simulink library "Renault_Library"  (Simulink 9.2)
settings: Solver=ode3  StartTime=0.0  StopTime=10.0  FixedStep=0.020  RelTol=1e-3  EnableMultiTasking=on
undefined symbols (not defined in the file or in its folder): ABC, AssystemProposal, b, chkval, CoeffA_C, CoeffB_C, CoeffMean_C, ConfTime_C, DiagDelay_C, DividePercent, FailureConfActive_C, FallingEdgeDuration_C, HighLevelThresholdVoltage_C, HighLevelVoltage_C, HighLevelVoltageTunable_C, InitValue_C, InputVoltRangeMax_C, InputVoltRangeMin_C, LowActiveContact_C, LowLevelThresholdVoltage_C, LowLevelVoltage_C, MaxOperatingVoltage_C, MinOperatingVoltage_C, mV2V_conversion, OUT1, OutOfRangeHigh_C, OutOfRangeLow_C, PinSupplySaturationMax, PinSupplySaturationMin, Res1Value_C, Res2Value_C, Res3Value_C, RisingEdgeDuration_C, RSA_CANSignalFailMonitoring, SampleTime_C, service14Table, service19Table, service22Table, service24Table, service28Table, service2ETable, service2FTable, service31Table, service87Table, SizeXStateDID2FTable, SizeXTable14, SizeXTable19, SizeXTable22, SizeXTable24, SizeXTable28, SizeXTable2E, SizeXTable2F, SizeXTable31, SizeXTable87, SizeYStateDID2FTable, SizeYTable14, SizeYTable19, SizeYTable22, SizeYTable24, SizeYTable28 ... (+22)
undefined symbols (not defined in the file): BatteryVoltage_ActivationThreshold_C, FrameStatus_AbsentValue_C, FrameStatus_ConfAbsentValue_C, MemThreshold_C, NAMEOFTHEMODEL_SAMPLE_TIME_CST, NbAbs1_C, NbConfAbs1_C, NbConfPres1_C, NbPres1_C, NOMDUSIGNAL_FaultConfTime_C, NOMDUSIGNAL_FaultRehabTime_C, NOMDUSIGNAL_switchvalue_C, NOMDUSIGNAL_unavailablevalue_C, PeriodFrame1_C, ResetTime_C, WakeUpTime_C
libraries: $bdroot x112, simulink x75
(part 25 of 32)

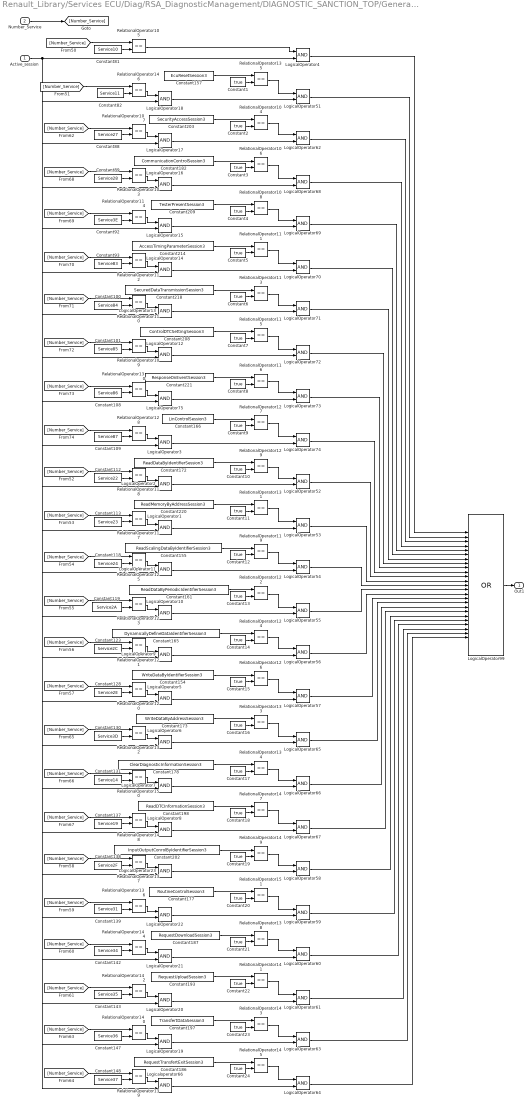
== system Renault_Library/Services ECU/Diag/RSA_DiagnosticManagement/DIAGNOSTIC_SANCTION_TOP/GeneralService/Service_Supported_In_Active_Session/SubsystemExtendedSession ==
  Active_session : Inport
  Number_Service : Inport  Port=2
  Constant{1..24} (24 alike) : Constant  Value=true  OutDataTypeStr=boolean  SampleTime=-1
  Constant100 : Constant  Value=Service84  OutDataTypeStr=Inherit: Inherit via back propagation  SampleTime=-1
  Constant101 : Constant  Value=Service85  OutDataTypeStr=Inherit: Inherit via back propagation  SampleTime=-1
  Constant108 : Constant  Value=Service86  OutDataTypeStr=Inherit: Inherit via back propagation  SampleTime=-1
  Constant109 : Constant  Value=Service87  OutDataTypeStr=Inherit: Inherit via back propagation  SampleTime=-1
  Constant112 : Constant  Value=Service22  OutDataTypeStr=Inherit: Inherit via back propagation  SampleTime=-1
  Constant113 : Constant  Value=Service23  OutDataTypeStr=Inherit: Inherit via back propagation  SampleTime=-1
  Constant118 : Constant  Value=Service24  OutDataTypeStr=Inherit: Inherit via back propagation  SampleTime=-1
  Constant119 : Constant  Value=Service2A  OutDataTypeStr=Inherit: Inherit via back propagation  SampleTime=-1
  Constant123 : Constant  Value=Service2C  OutDataTypeStr=Inherit: Inherit via back propagation  SampleTime=-1
  Constant128 : Constant  Value=Service2E  OutDataTypeStr=Inherit: Inherit via back propagation  SampleTime=-1
  Constant130 : Constant  Value=Service3D  OutDataTypeStr=Inherit: Inherit via back propagation  SampleTime=-1
  Constant131 : Constant  Value=Service14  OutDataTypeStr=Inherit: Inherit via back propagation  SampleTime=-1
  Constant137 : Constant  Value=Service19  OutDataTypeStr=Inherit: Inherit via back propagation  SampleTime=-1
  Constant138 : Constant  Value=Service2F  OutDataTypeStr=Inherit: Inherit via back propagation  SampleTime=-1
  Constant139 : Constant  Value=Service31  OutDataTypeStr=Inherit: Inherit via back propagation  SampleTime=-1
  Constant142 : Constant  Value=Service34  OutDataTypeStr=Inherit: Inherit via back propagation  SampleTime=-1
  Constant143 : Constant  Value=Service35  OutDataTypeStr=Inherit: Inherit via back propagation  SampleTime=-1
  Constant147 : Constant  Value=Service36  OutDataTypeStr=Inherit: Inherit via back propagation  SampleTime=-1
  Constant148 : Constant  Value=Service37  OutDataTypeStr=Inherit: Inherit via back propagation  SampleTime=-1
  Constant154 : Constant  Value=WriteDataByIdentifierSession3  OutDataTypeStr=Inherit: Inherit via back propagation  SampleTime=-1
  Constant155 : Constant  Value=ReadScalingDataByIdentifierSession3  OutDataTypeStr=Inherit: Inherit via back propagation  SampleTime=-1
  Constant157 : Constant  Value=EcuResetSession3  OutDataTypeStr=Inherit: Inherit via back propagation  SampleTime=-1
  Constant161 : Constant  Value=ReadDataByPeriodicIdentifierSession3  OutDataTypeStr=Inherit: Inherit via back propagation  SampleTime=-1
  Constant165 : Constant  Value=DynamicallyDefineDataIdentifierSession3  OutDataTypeStr=Inherit: Inherit via back propagation  SampleTime=-1
  Constant166 : Constant  Value=LinControlSession3  OutDataTypeStr=Inherit: Inherit via back propagation  SampleTime=-1
  Constant172 : Constant  Value=ReadDataByIdentifierSession3  OutDataTypeStr=Inherit: Inherit via back propagation  SampleTime=-1
  Constant173 : Constant  Value=WriteDataByAddressSession3  OutDataTypeStr=Inherit: Inherit via back propagation  SampleTime=-1
  Constant177 : Constant  Value=RoutineControlSession3  OutDataTypeStr=Inherit: Inherit via back propagation  SampleTime=-1
  Constant178 : Constant  Value=ClearDiagnosticInformationSession3  OutDataTypeStr=Inherit: Inherit via back propagation  SampleTime=-1
  Constant182 : Constant  Value=CommunicationControlSession3  OutDataTypeStr=Inherit: Inherit via back propagation  SampleTime=-1
  Constant186 : Constant  Value=RequestTransfertExitSession3  OutDataTypeStr=Inherit: Inherit via back propagation  SampleTime=-1
  Constant187 : Constant  Value=RequestDownloadSession3  OutDataTypeStr=Inherit: Inherit via back propagation  SampleTime=-1
  Constant193 : Constant  Value=RequestUploadSession3  OutDataTypeStr=Inherit: Inherit via back propagation  SampleTime=-1
  Constant197 : Constant  Value=TransfertDataSession3  OutDataTypeStr=Inherit: Inherit via back propagation  SampleTime=-1
  Constant198 : Constant  Value=ReadDTCInformationSession3  OutDataTypeStr=Inherit: Inherit via back propagation  SampleTime=-1
  Constant202 : Constant  Value=InputOutputConrolByIdentifierSession3  OutDataTypeStr=Inherit: Inherit via back propagation  SampleTime=-1
  Constant203 : Constant  Value=SecurityAccessSession3  OutDataTypeStr=Inherit: Inherit via back propagation  SampleTime=-1
  Constant208 : Constant  Value=ControlDTCSettingSession3  OutDataTypeStr=Inherit: Inherit via back propagation  SampleTime=-1
  Constant209 : Constant  Value=TesterPresentSession3  OutDataTypeStr=Inherit: Inherit via back propagation  SampleTime=-1
  Constant214 : Constant  Value=AccessTimingParameterSession3  OutDataTypeStr=Inherit: Inherit via back propagation  SampleTime=-1
  Constant218 : Constant  Value=SecuredDataTransmissionSession3  OutDataTypeStr=Inherit: Inherit via back propagation  SampleTime=-1
  Constant220 : Constant  Value=ReadMemoryByAddressSession3  OutDataTypeStr=Inherit: Inherit via back propagation  SampleTime=-1
  Constant221 : Constant  Value=ResponseOnEventSession3  OutDataTypeStr=Inherit: Inherit via back propagation  SampleTime=-1
  Constant81 : Constant  Value=Service10  OutDataTypeStr=Inherit: Inherit via back propagation  SampleTime=-1
  Constant82 : Constant  Value=Service11  OutDataTypeStr=Inherit: Inherit via back propagation  SampleTime=-1
  Constant88 : Constant  Value=Service27  OutDataTypeStr=Inherit: Inherit via back propagation  SampleTime=-1
  Constant89 : Constant  Value=Service28  OutDataTypeStr=Inherit: Inherit via back propagation  SampleTime=-1
  Constant92 : Constant  Value=Service3E  OutDataTypeStr=Inherit: Inherit via back propagation  SampleTime=-1
  Constant93 : Constant  Value=Service83  OutDataTypeStr=Inherit: Inherit via back propagation  SampleTime=-1
  LogicalOperator{1..23,51..75} (48 alike) : Logic  OutDataTypeStr=boolean
  LogicalOperator99 : Logic  Operator=OR  Inputs=25  OutDataTypeStr=boolean
  Logicaloperator66 : Logic  OutDataTypeStr=boolean
  RelationalOperator{103..151} (49 alike) : RelationalOperator  Operator===  OutDataTypeStr=boolean  RndMeth=Nearest  InputSameDT=on
  Out1 : Outport
  wires:
  Constant81/1 -> RelationalOperator105/2
  Active_session/1 -> LogicalOperator19/2, LogicalOperator20/2, LogicalOperator21/2, LogicalOperator22/2, LogicalOperator23/2, LogicalOperator8/2, LogicalOperator7/2, Logicaloperator66/2, LogicalOperator6/2, LogicalOperator5/2, LogicalOperator9/2, LogicalOperator10/2, LogicalOperator11/2, LogicalOperator1/2, LogicalOperator2/2, LogicalOperator3/2, LogicalOperator75/2, LogicalOperator12/2, LogicalOperator13/2, LogicalOperator14/2, LogicalOperator15/2, LogicalOperator16/2, LogicalOperator17/2, LogicalOperator18/2, LogicalOperator4/2
  RelationalOperator105/1 -> LogicalOperator4/1
  [Number_Service] -> RelationalOperator105/1
  Constant82/1 -> RelationalOperator146/2
  Constant157/1 -> RelationalOperator135/1
  Constant1/1 -> RelationalOperator135/2
  RelationalOperator135/1 -> LogicalOperator51/1
  LogicalOperator18/1 -> LogicalOperator51/2
  RelationalOperator146/1 -> LogicalOperator18/1
  [Number_Service] -> RelationalOperator146/1
  Constant88/1 -> RelationalOperator107/2
  Constant203/1 -> RelationalOperator104/1
  Constant2/1 -> RelationalOperator104/2
  RelationalOperator104/1 -> LogicalOperator62/1
  LogicalOperator17/1 -> LogicalOperator62/2
  RelationalOperator107/1 -> LogicalOperator17/1
  [Number_Service] -> RelationalOperator107/1
  Constant89/1 -> RelationalOperator103/2
  Constant182/1 -> RelationalOperator106/1
  Constant3/1 -> RelationalOperator106/2
  RelationalOperator106/1 -> LogicalOperator68/1
  LogicalOperator16/1 -> LogicalOperator68/2
  RelationalOperator103/1 -> LogicalOperator16/1
  [Number_Service] -> RelationalOperator103/1
  Constant92/1 -> RelationalOperator114/2
  Constant209/1 -> RelationalOperator108/1
  Constant4/1 -> RelationalOperator108/2
  RelationalOperator108/1 -> LogicalOperator69/1
  LogicalOperator15/1 -> LogicalOperator69/2
  RelationalOperator114/1 -> LogicalOperator15/1
  [Number_Service] -> RelationalOperator114/1
  Constant93/1 -> RelationalOperator112/2
  Constant214/1 -> RelationalOperator111/1
  Constant5/1 -> RelationalOperator111/2
  RelationalOperator111/1 -> LogicalOperator70/1
  LogicalOperator14/1 -> LogicalOperator70/2
  RelationalOperator112/1 -> LogicalOperator14/1
  [Number_Service] -> RelationalOperator112/1
  Constant100/1 -> RelationalOperator110/2
  Constant218/1 -> RelationalOperator113/1
  Constant6/1 -> RelationalOperator113/2
  RelationalOperator113/1 -> LogicalOperator71/1
  LogicalOperator13/1 -> LogicalOperator71/2
  RelationalOperator110/1 -> LogicalOperator13/1
  [Number_Service] -> RelationalOperator110/1
  Constant101/1 -> RelationalOperator109/2
  Constant208/1 -> RelationalOperator115/1
  Constant7/1 -> RelationalOperator115/2
  RelationalOperator115/1 -> LogicalOperator72/1
  LogicalOperator12/1 -> LogicalOperator72/2
  RelationalOperator109/1 -> LogicalOperator12/1
  [Number_Service] -> RelationalOperator109/1
  Constant108/1 -> RelationalOperator130/2
  Constant221/1 -> RelationalOperator116/1
  Constant8/1 -> RelationalOperator116/2
  RelationalOperator116/1 -> LogicalOperator73/1
  LogicalOperator75/1 -> LogicalOperator73/2
  RelationalOperator130/1 -> LogicalOperator75/1
  [Number_Service] -> RelationalOperator130/1
  Constant109/1 -> RelationalOperator128/2
  Constant166/1 -> RelationalOperator127/1
  Constant9/1 -> RelationalOperator127/2
  RelationalOperator127/1 -> LogicalOperator74/1
  LogicalOperator3/1 -> LogicalOperator74/2
  RelationalOperator128/1 -> LogicalOperator3/1
  [Number_Service] -> RelationalOperator128/1
  Constant112/1 -> RelationalOperator118/2
  Constant172/1 -> RelationalOperator129/1
  Constant10/1 -> RelationalOperator129/2
  RelationalOperator129/1 -> LogicalOperator52/1
  LogicalOperator2/1 -> LogicalOperator52/2
  RelationalOperator118/1 -> LogicalOperator2/1
  [Number_Service] -> RelationalOperator118/1
  Constant113/1 -> RelationalOperator117/2
  Constant220/1 -> RelationalOperator131/1
  Constant11/1 -> RelationalOperator131/2
  RelationalOperator131/1 -> LogicalOperator53/1
  LogicalOperator1/1 -> LogicalOperator53/2
  RelationalOperator117/1 -> LogicalOperator1/1
  [Number_Service] -> RelationalOperator117/1
  Constant118/1 -> RelationalOperator125/2
  Constant155/1 -> RelationalOperator119/1
  RelationalOperator119/1 -> LogicalOperator54/1
  LogicalOperator11/1 -> LogicalOperator54/2
  RelationalOperator125/1 -> LogicalOperator11/1
  [Number_Service] -> RelationalOperator125/1
  Constant119/1 -> RelationalOperator123/2
  Constant161/1 -> RelationalOperator122/1
  Constant13/1 -> RelationalOperator122/2
  RelationalOperator122/1 -> LogicalOperator55/1
  LogicalOperator10/1 -> LogicalOperator55/2
  RelationalOperator123/1 -> LogicalOperator10/1
  [Number_Service] -> RelationalOperator123/1
  Constant123/1 -> RelationalOperator121/2
  Constant165/1 -> RelationalOperator124/1
  Constant14/1 -> RelationalOperator124/2
  RelationalOperator124/1 -> LogicalOperator56/1
  LogicalOperator9/1 -> LogicalOperator56/2
  RelationalOperator121/1 -> LogicalOperator9/1
  [Number_Service] -> RelationalOperator121/1
  Constant128/1 -> RelationalOperator120/2
  Constant154/1 -> RelationalOperator126/1
  Constant15/1 -> RelationalOperator126/2
  RelationalOperator126/1 -> LogicalOperator57/1
  LogicalOperator5/1 -> LogicalOperator57/2
  RelationalOperator120/1 -> LogicalOperator5/1
  [Number_Service] -> RelationalOperator120/1
  Constant130/1 -> RelationalOperator132/2
  Constant173/1 -> RelationalOperator133/1
  Constant16/1 -> RelationalOperator133/2
  RelationalOperator133/1 -> LogicalOperator65/1
  LogicalOperator6/1 -> LogicalOperator65/2
  RelationalOperator132/1 -> LogicalOperator6/1
  [Number_Service] -> RelationalOperator132/1
  Constant131/1 -> RelationalOperator150/2
  Constant178/1 -> RelationalOperator134/1
  Constant17/1 -> RelationalOperator134/2
  RelationalOperator134/1 -> LogicalOperator66/1
  LogicalOperator7/1 -> LogicalOperator66/2
  RelationalOperator150/1 -> LogicalOperator7/1
  [Number_Service] -> RelationalOperator150/1
  Constant137/1 -> RelationalOperator148/2
  Constant198/1 -> RelationalOperator147/1
  Constant18/1 -> RelationalOperator147/2
  RelationalOperator147/1 -> LogicalOperator67/1
  LogicalOperator8/1 -> LogicalOperator67/2
  RelationalOperator148/1 -> LogicalOperator8/1
  [Number_Service] -> RelationalOperator148/1
  Constant138/1 -> RelationalOperator137/2
  Constant202/1 -> RelationalOperator149/1
  Constant19/1 -> RelationalOperator149/2
  RelationalOperator149/1 -> LogicalOperator58/1
  LogicalOperator23/1 -> LogicalOperator58/2
  RelationalOperator137/1 -> LogicalOperator23/1
  [Number_Service] -> RelationalOperator137/1
  Constant139/1 -> RelationalOperator136/2
  Constant177/1 -> RelationalOperator151/1
  Constant20/1 -> RelationalOperator151/2
  RelationalOperator151/1 -> LogicalOperator59/1
  LogicalOperator22/1 -> LogicalOperator59/2
  RelationalOperator136/1 -> LogicalOperator22/1
  [Number_Service] -> RelationalOperator136/1
  Constant142/1 -> RelationalOperator144/2
  Constant187/1 -> RelationalOperator138/1
  Constant21/1 -> RelationalOperator138/2
  RelationalOperator138/1 -> LogicalOperator60/1
  LogicalOperator21/1 -> LogicalOperator60/2
  RelationalOperator144/1 -> LogicalOperator21/1
  [Number_Service] -> RelationalOperator144/1
  Constant143/1 -> RelationalOperator142/2
  Constant193/1 -> RelationalOperator141/1
  Constant22/1 -> RelationalOperator141/2
  RelationalOperator141/1 -> LogicalOperator61/1
  LogicalOperator20/1 -> LogicalOperator61/2
  RelationalOperator142/1 -> LogicalOperator20/1
  [Number_Service] -> RelationalOperator142/1
  Constant147/1 -> RelationalOperator140/2
  Constant197/1 -> RelationalOperator143/1
  Constant23/1 -> RelationalOperator143/2
  RelationalOperator143/1 -> LogicalOperator63/1
  LogicalOperator19/1 -> LogicalOperator63/2
  RelationalOperator140/1 -> LogicalOperator19/1
  [Number_Service] -> RelationalOperator140/1
  Constant148/1 -> RelationalOperator139/2
  Constant186/1 -> RelationalOperator145/1
  Constant24/1 -> RelationalOperator145/2
  RelationalOperator145/1 -> LogicalOperator64/1
  Logicaloperator66/1 -> LogicalOperator64/2
  RelationalOperator139/1 -> Logicaloperator66/1
  [Number_Service] -> RelationalOperator139/1
  LogicalOperator4/1 -> LogicalOperator99/1
  LogicalOperator51/1 -> LogicalOperator99/2
  LogicalOperator62/1 -> LogicalOperator99/3
  LogicalOperator68/1 -> LogicalOperator99/4
  LogicalOperator69/1 -> LogicalOperator99/5
  LogicalOperator70/1 -> LogicalOperator99/6
  LogicalOperator71/1 -> LogicalOperator99/7
  LogicalOperator72/1 -> LogicalOperator99/8
  LogicalOperator73/1 -> LogicalOperator99/9
  LogicalOperator74/1 -> LogicalOperator99/10
  LogicalOperator52/1 -> LogicalOperator99/11
  LogicalOperator53/1 -> LogicalOperator99/12
  LogicalOperator54/1 -> LogicalOperator99/13
  LogicalOperator55/1 -> LogicalOperator99/14
  LogicalOperator56/1 -> LogicalOperator99/15
  LogicalOperator57/1 -> LogicalOperator99/16
  LogicalOperator65/1 -> LogicalOperator99/17
  LogicalOperator66/1 -> LogicalOperator99/18
  LogicalOperator67/1 -> LogicalOperator99/19
  LogicalOperator58/1 -> LogicalOperator99/20
  LogicalOperator59/1 -> LogicalOperator99/21
  LogicalOperator60/1 -> LogicalOperator99/22
  LogicalOperator61/1 -> LogicalOperator99/23
  LogicalOperator63/1 -> LogicalOperator99/24
  LogicalOperator64/1 -> LogicalOperator99/25
  LogicalOperator99/1 -> Out1/1
  Number_Service/1 -> [Number_Service]
  Constant12/1 -> RelationalOperator119/2

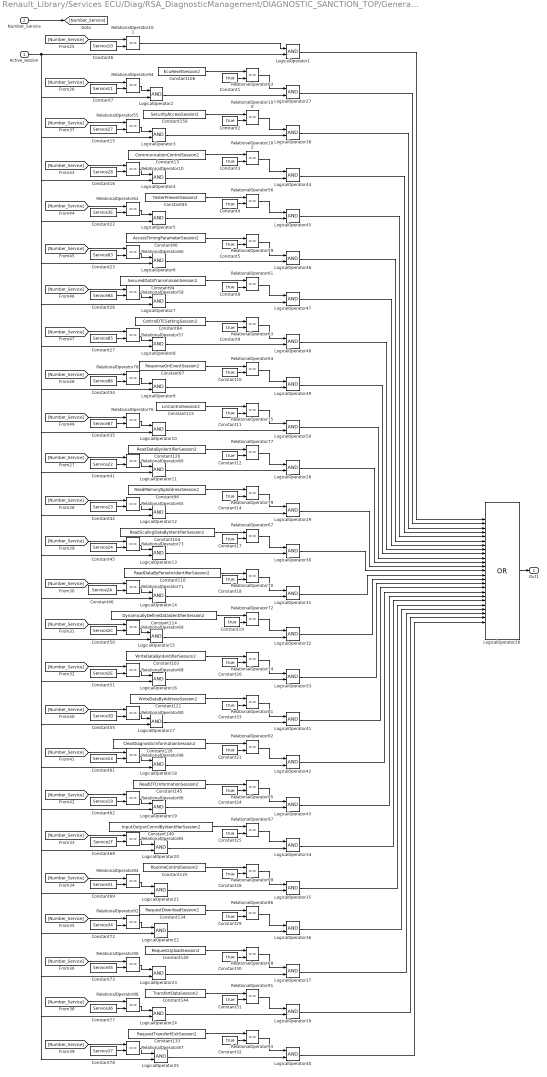
== system Renault_Library/Services ECU/Diag/RSA_DiagnosticManagement/DIAGNOSTIC_SANCTION_TOP/GeneralService/Service_Supported_In_Active_Session/SubsystemProgrammingSession ==
  Active_session : Inport
  Number_Service : Inport  Port=2
  Constant{1..5,8..12,14,17..21,24..25,28..33} (24 alike) : Constant  Value=true  OutDataTypeStr=boolean  SampleTime=-1
  Constant103 : Constant  Value=WriteDataByIdentifierSession2  OutDataTypeStr=Inherit: Inherit via back propagation  SampleTime=-1
  Constant104 : Constant  Value=ReadScalingDataByIdentifierSession2  OutDataTypeStr=Inherit: Inherit via back propagation  SampleTime=-1
  Constant106 : Constant  Value=EcuResetSession2  OutDataTypeStr=Inherit: Inherit via back propagation  SampleTime=-1
  Constant110 : Constant  Value=ReadDataByPeriodicIdentifierSession2  OutDataTypeStr=Inherit: Inherit via back propagation  SampleTime=-1
  Constant114 : Constant  Value=DynamicallyDefineDataIdentifierSession2  OutDataTypeStr=Inherit: Inherit via back propagation  SampleTime=-1
  Constant115 : Constant  Value=LinControlSession2  OutDataTypeStr=Inherit: Inherit via back propagation  SampleTime=-1
  Constant120 : Constant  Value=ReadDataByIdentifierSession2  OutDataTypeStr=Inherit: Inherit via back propagation  SampleTime=-1
  Constant121 : Constant  Value=WriteDataByAddressSession2  OutDataTypeStr=Inherit: Inherit via back propagation  SampleTime=-1
  Constant125 : Constant  Value=RoutineControlSession2  OutDataTypeStr=Inherit: Inherit via back propagation  SampleTime=-1
  Constant126 : Constant  Value=ClearDiagnosticInformationSession2  OutDataTypeStr=Inherit: Inherit via back propagation  SampleTime=-1
  Constant13 : Constant  Value=CommunicationControlSession2  OutDataTypeStr=Inherit: Inherit via back propagation  SampleTime=-1
  Constant133 : Constant  Value=RequestTransfertExitSession2  OutDataTypeStr=Inherit: Inherit via back propagation  SampleTime=-1
  Constant134 : Constant  Value=RequestDownloadSession2  OutDataTypeStr=Inherit: Inherit via back propagation  SampleTime=-1
  Constant140 : Constant  Value=RequestUploadSession2  OutDataTypeStr=Inherit: Inherit via back propagation  SampleTime=-1
  Constant144 : Constant  Value=TransfertDataSession2  OutDataTypeStr=Inherit: Inherit via back propagation  SampleTime=-1
  Constant145 : Constant  Value=ReadDTCInformationSession2  OutDataTypeStr=Inherit: Inherit via back propagation  SampleTime=-1
  Constant149 : Constant  Value=InputOutputConrolByIdentifierSession2  OutDataTypeStr=Inherit: Inherit via back propagation  SampleTime=-1
  Constant15 : Constant  Value=Service27  OutDataTypeStr=Inherit: Inherit via back propagation  SampleTime=-1
  Constant150 : Constant  Value=SecurityAccessSession2  OutDataTypeStr=Inherit: Inherit via back propagation  SampleTime=-1
  Constant16 : Constant  Value=Service28  OutDataTypeStr=Inherit: Inherit via back propagation  SampleTime=-1
  Constant22 : Constant  Value=Service3E  OutDataTypeStr=Inherit: Inherit via back propagation  SampleTime=-1
  Constant23 : Constant  Value=Service83  OutDataTypeStr=Inherit: Inherit via back propagation  SampleTime=-1
  Constant26 : Constant  Value=Service84  OutDataTypeStr=Inherit: Inherit via back propagation  SampleTime=-1
  Constant27 : Constant  Value=Service85  OutDataTypeStr=Inherit: Inherit via back propagation  SampleTime=-1
  Constant34 : Constant  Value=Service86  OutDataTypeStr=Inherit: Inherit via back propagation  SampleTime=-1
  Constant35 : Constant  Value=Service87  OutDataTypeStr=Inherit: Inherit via back propagation  SampleTime=-1
  Constant41 : Constant  Value=Service22  OutDataTypeStr=Inherit: Inherit via back propagation  SampleTime=-1
  Constant42 : Constant  Value=Service23  OutDataTypeStr=Inherit: Inherit via back propagation  SampleTime=-1
  Constant45 : Constant  Value=Service24  OutDataTypeStr=Inherit: Inherit via back propagation  SampleTime=-1
  Constant46 : Constant  Value=Service2A  OutDataTypeStr=Inherit: Inherit via back propagation  SampleTime=-1
  Constant50 : Constant  Value=Service2C  OutDataTypeStr=Inherit: Inherit via back propagation  SampleTime=-1
  Constant51 : Constant  Value=Service2E  OutDataTypeStr=Inherit: Inherit via back propagation  SampleTime=-1
  Constant55 : Constant  Value=Service3D  OutDataTypeStr=Inherit: Inherit via back propagation  SampleTime=-1
  Constant6 : Constant  Value=Service10  OutDataTypeStr=Inherit: Inherit via back propagation  SampleTime=-1
  Constant61 : Constant  Value=Service14  OutDataTypeStr=Inherit: Inherit via back propagation  SampleTime=-1
  Constant62 : Constant  Value=Service19  OutDataTypeStr=Inherit: Inherit via back propagation  SampleTime=-1
  Constant68 : Constant  Value=Service2F  OutDataTypeStr=Inherit: Inherit via back propagation  SampleTime=-1
  Constant69 : Constant  Value=Service31  OutDataTypeStr=Inherit: Inherit via back propagation  SampleTime=-1
  Constant7 : Constant  Value=Service11  OutDataTypeStr=Inherit: Inherit via back propagation  SampleTime=-1
  Constant72 : Constant  Value=Service34  OutDataTypeStr=Inherit: Inherit via back propagation  SampleTime=-1
  Constant73 : Constant  Value=Service35  OutDataTypeStr=Inherit: Inherit via back propagation  SampleTime=-1
  Constant77 : Constant  Value=Service36  OutDataTypeStr=Inherit: Inherit via back propagation  SampleTime=-1
  Constant78 : Constant  Value=Service37  OutDataTypeStr=Inherit: Inherit via back propagation  SampleTime=-1
  Constant84 : Constant  Value=ControlDTCSettingSession2  OutDataTypeStr=Inherit: Inherit via back propagation  SampleTime=-1
  Constant85 : Constant  Value=TesterPresentSession2  OutDataTypeStr=Inherit: Inherit via back propagation  SampleTime=-1
  Constant90 : Constant  Value=AccessTimingParameterSession2  OutDataTypeStr=Inherit: Inherit via back propagation  SampleTime=-1
  Constant94 : Constant  Value=SecuredDataTransmissionSession2  OutDataTypeStr=Inherit: Inherit via back propagation  SampleTime=-1
  Constant96 : Constant  Value=ReadMemoryByAddressSession2  OutDataTypeStr=Inherit: Inherit via back propagation  SampleTime=-1
  Constant97 : Constant  Value=ResponseOnEventSession2  OutDataTypeStr=Inherit: Inherit via back propagation  SampleTime=-1
  LogicalOperator{1..25,27..50} (49 alike) : Logic  OutDataTypeStr=boolean
  LogicalOperator26 : Logic  Operator=OR  Inputs=25  OutDataTypeStr=boolean
  RelationalOperator{10,55..102} (49 alike) : RelationalOperator  Operator===  OutDataTypeStr=boolean  RndMeth=Nearest  InputSameDT=on
  Out1 : Outport
  wires:
  Constant6/1 -> RelationalOperator101/2
  Active_session/1 -> LogicalOperator11/2, LogicalOperator25/2, LogicalOperator17/2, LogicalOperator18/2, LogicalOperator19/2, LogicalOperator20/2, LogicalOperator21/2, LogicalOperator22/2, LogicalOperator23/2, LogicalOperator24/2, LogicalOperator9/2, LogicalOperator10/2, LogicalOperator12/2, LogicalOperator13/2, LogicalOperator14/2, LogicalOperator15/2, LogicalOperator16/2, LogicalOperator1/2, LogicalOperator2/2, LogicalOperator3/2, LogicalOperator4/2, LogicalOperator5/2, LogicalOperator6/2, LogicalOperator7/2, LogicalOperator8/2
  RelationalOperator101/1 -> LogicalOperator1/1
  [Number_Service] -> RelationalOperator101/1
  Constant7/1 -> RelationalOperator94/2
  Constant106/1 -> RelationalOperator83/1
  Constant1/1 -> RelationalOperator83/2
  RelationalOperator83/1 -> LogicalOperator27/1
  LogicalOperator2/1 -> LogicalOperator27/2
  RelationalOperator94/1 -> LogicalOperator2/1
  [Number_Service] -> RelationalOperator94/1
  Constant15/1 -> RelationalOperator55/2
  Constant150/1 -> RelationalOperator100/1
  Constant2/1 -> RelationalOperator100/2
  RelationalOperator100/1 -> LogicalOperator38/1
  LogicalOperator3/1 -> LogicalOperator38/2
  RelationalOperator55/1 -> LogicalOperator3/1
  [Number_Service] -> RelationalOperator55/1
  Constant16/1 -> RelationalOperator10/2
  Constant13/1 -> RelationalOperator102/1
  Constant3/1 -> RelationalOperator102/2
  RelationalOperator102/1 -> LogicalOperator44/1
  LogicalOperator4/1 -> LogicalOperator44/2
  RelationalOperator10/1 -> LogicalOperator4/1
  [Number_Service] -> RelationalOperator10/1
  Constant22/1 -> RelationalOperator62/2
  Constant85/1 -> RelationalOperator56/1
  Constant4/1 -> RelationalOperator56/2
  RelationalOperator56/1 -> LogicalOperator45/1
  LogicalOperator5/1 -> LogicalOperator45/2
  RelationalOperator62/1 -> LogicalOperator5/1
  [Number_Service] -> RelationalOperator62/1
  Constant23/1 -> RelationalOperator60/2
  Constant90/1 -> RelationalOperator59/1
  Constant5/1 -> RelationalOperator59/2
  RelationalOperator59/1 -> LogicalOperator46/1
  LogicalOperator6/1 -> LogicalOperator46/2
  RelationalOperator60/1 -> LogicalOperator6/1
  [Number_Service] -> RelationalOperator60/1
  Constant26/1 -> RelationalOperator58/2
  Constant94/1 -> RelationalOperator61/1
  Constant8/1 -> RelationalOperator61/2
  RelationalOperator61/1 -> LogicalOperator47/1
  LogicalOperator7/1 -> LogicalOperator47/2
  RelationalOperator58/1 -> LogicalOperator7/1
  [Number_Service] -> RelationalOperator58/1
  Constant27/1 -> RelationalOperator57/2
  Constant84/1 -> RelationalOperator63/1
  Constant9/1 -> RelationalOperator63/2
  RelationalOperator63/1 -> LogicalOperator48/1
  LogicalOperator8/1 -> LogicalOperator48/2
  RelationalOperator57/1 -> LogicalOperator8/1
  [Number_Service] -> RelationalOperator57/1
  Constant34/1 -> RelationalOperator78/2
  Constant97/1 -> RelationalOperator64/1
  Constant10/1 -> RelationalOperator64/2
  RelationalOperator64/1 -> LogicalOperator49/1
  LogicalOperator9/1 -> LogicalOperator49/2
  RelationalOperator78/1 -> LogicalOperator9/1
  [Number_Service] -> RelationalOperator78/1
  Constant35/1 -> RelationalOperator76/2
  Constant115/1 -> RelationalOperator75/1
  Constant11/1 -> RelationalOperator75/2
  RelationalOperator75/1 -> LogicalOperator50/1
  LogicalOperator10/1 -> LogicalOperator50/2
  RelationalOperator76/1 -> LogicalOperator10/1
  [Number_Service] -> RelationalOperator76/1
  Constant41/1 -> RelationalOperator66/2
  Constant120/1 -> RelationalOperator77/1
  Constant12/1 -> RelationalOperator77/2
  RelationalOperator77/1 -> LogicalOperator28/1
  LogicalOperator11/1 -> LogicalOperator28/2
  RelationalOperator66/1 -> LogicalOperator11/1
  [Number_Service] -> RelationalOperator66/1
  Constant42/1 -> RelationalOperator65/2
  Constant96/1 -> RelationalOperator79/1
  Constant14/1 -> RelationalOperator79/2
  RelationalOperator79/1 -> LogicalOperator29/1
  LogicalOperator12/1 -> LogicalOperator29/2
  RelationalOperator65/1 -> LogicalOperator12/1
  [Number_Service] -> RelationalOperator65/1
  Constant45/1 -> RelationalOperator73/2
  Constant104/1 -> RelationalOperator67/1
  Constant17/1 -> RelationalOperator67/2
  RelationalOperator67/1 -> LogicalOperator30/1
  LogicalOperator13/1 -> LogicalOperator30/2
  RelationalOperator73/1 -> LogicalOperator13/1
  [Number_Service] -> RelationalOperator73/1
  Constant46/1 -> RelationalOperator71/2
  Constant110/1 -> RelationalOperator70/1
  Constant18/1 -> RelationalOperator70/2
  RelationalOperator70/1 -> LogicalOperator31/1
  LogicalOperator14/1 -> LogicalOperator31/2
  RelationalOperator71/1 -> LogicalOperator14/1
  [Number_Service] -> RelationalOperator71/1
  Constant50/1 -> RelationalOperator69/2
  Constant114/1 -> RelationalOperator72/1
  Constant19/1 -> RelationalOperator72/2
  RelationalOperator72/1 -> LogicalOperator32/1
  LogicalOperator15/1 -> LogicalOperator32/2
  RelationalOperator69/1 -> LogicalOperator15/1
  [Number_Service] -> RelationalOperator69/1
  Constant51/1 -> RelationalOperator68/2
  Constant103/1 -> RelationalOperator74/1
  Constant20/1 -> RelationalOperator74/2
  RelationalOperator74/1 -> LogicalOperator33/1
  LogicalOperator16/1 -> LogicalOperator33/2
  RelationalOperator68/1 -> LogicalOperator16/1
  [Number_Service] -> RelationalOperator68/1
  Constant55/1 -> RelationalOperator80/2
  Constant121/1 -> RelationalOperator81/1
  Constant33/1 -> RelationalOperator81/2
  RelationalOperator81/1 -> LogicalOperator41/1
  LogicalOperator17/1 -> LogicalOperator41/2
  RelationalOperator80/1 -> LogicalOperator17/1
  [Number_Service] -> RelationalOperator80/1
  Constant61/1 -> RelationalOperator98/2
  Constant126/1 -> RelationalOperator82/1
  Constant21/1 -> RelationalOperator82/2
  RelationalOperator82/1 -> LogicalOperator42/1
  LogicalOperator18/1 -> LogicalOperator42/2
  RelationalOperator98/1 -> LogicalOperator18/1
  [Number_Service] -> RelationalOperator98/1
  Constant62/1 -> RelationalOperator96/2
  Constant145/1 -> RelationalOperator95/1
  Constant24/1 -> RelationalOperator95/2
  RelationalOperator95/1 -> LogicalOperator43/1
  LogicalOperator19/1 -> LogicalOperator43/2
  RelationalOperator96/1 -> LogicalOperator19/1
  [Number_Service] -> RelationalOperator96/1
  Constant68/1 -> RelationalOperator85/2
  Constant149/1 -> RelationalOperator97/1
  Constant25/1 -> RelationalOperator97/2
  RelationalOperator97/1 -> LogicalOperator34/1
  LogicalOperator20/1 -> LogicalOperator34/2
  RelationalOperator85/1 -> LogicalOperator20/1
  [Number_Service] -> RelationalOperator85/1
  Constant69/1 -> RelationalOperator84/2
  Constant125/1 -> RelationalOperator99/1
  Constant28/1 -> RelationalOperator99/2
  RelationalOperator99/1 -> LogicalOperator35/1
  LogicalOperator21/1 -> LogicalOperator35/2
  RelationalOperator84/1 -> LogicalOperator21/1
  [Number_Service] -> RelationalOperator84/1
  Constant72/1 -> RelationalOperator92/2
  Constant134/1 -> RelationalOperator86/1
  Constant29/1 -> RelationalOperator86/2
  RelationalOperator86/1 -> LogicalOperator36/1
  LogicalOperator22/1 -> LogicalOperator36/2
  RelationalOperator92/1 -> LogicalOperator22/1
  [Number_Service] -> RelationalOperator92/1
  Constant73/1 -> RelationalOperator90/2
  Constant140/1 -> RelationalOperator89/1
  Constant30/1 -> RelationalOperator89/2
  RelationalOperator89/1 -> LogicalOperator37/1
  LogicalOperator23/1 -> LogicalOperator37/2
  RelationalOperator90/1 -> LogicalOperator23/1
  [Number_Service] -> RelationalOperator90/1
  Constant77/1 -> RelationalOperator88/2
  Constant144/1 -> RelationalOperator91/1
  Constant31/1 -> RelationalOperator91/2
  RelationalOperator91/1 -> LogicalOperator39/1
  LogicalOperator24/1 -> LogicalOperator39/2
  RelationalOperator88/1 -> LogicalOperator24/1
  [Number_Service] -> RelationalOperator88/1
  Constant78/1 -> RelationalOperator87/2
  Constant133/1 -> RelationalOperator93/1
  Constant32/1 -> RelationalOperator93/2
  RelationalOperator93/1 -> LogicalOperator40/1
  LogicalOperator25/1 -> LogicalOperator40/2
  RelationalOperator87/1 -> LogicalOperator25/1
  [Number_Service] -> RelationalOperator87/1
  LogicalOperator1/1 -> LogicalOperator26/1
  LogicalOperator27/1 -> LogicalOperator26/2
  LogicalOperator38/1 -> LogicalOperator26/3
  LogicalOperator44/1 -> LogicalOperator26/4
  LogicalOperator45/1 -> LogicalOperator26/5
  LogicalOperator46/1 -> LogicalOperator26/6
  LogicalOperator47/1 -> LogicalOperator26/7
  LogicalOperator48/1 -> LogicalOperator26/8
  LogicalOperator49/1 -> LogicalOperator26/9
  LogicalOperator50/1 -> LogicalOperator26/10
  LogicalOperator28/1 -> LogicalOperator26/11
  LogicalOperator29/1 -> LogicalOperator26/12
  LogicalOperator30/1 -> LogicalOperator26/13
  LogicalOperator31/1 -> LogicalOperator26/14
  LogicalOperator32/1 -> LogicalOperator26/15
  LogicalOperator33/1 -> LogicalOperator26/16
  LogicalOperator41/1 -> LogicalOperator26/17
  LogicalOperator42/1 -> LogicalOperator26/18
  LogicalOperator43/1 -> LogicalOperator26/19
  LogicalOperator34/1 -> LogicalOperator26/20
  LogicalOperator35/1 -> LogicalOperator26/21
  LogicalOperator36/1 -> LogicalOperator26/22
  LogicalOperator37/1 -> LogicalOperator26/23
  LogicalOperator39/1 -> LogicalOperator26/24
  LogicalOperator40/1 -> LogicalOperator26/25
  LogicalOperator26/1 -> Out1/1
  Number_Service/1 -> [Number_Service]

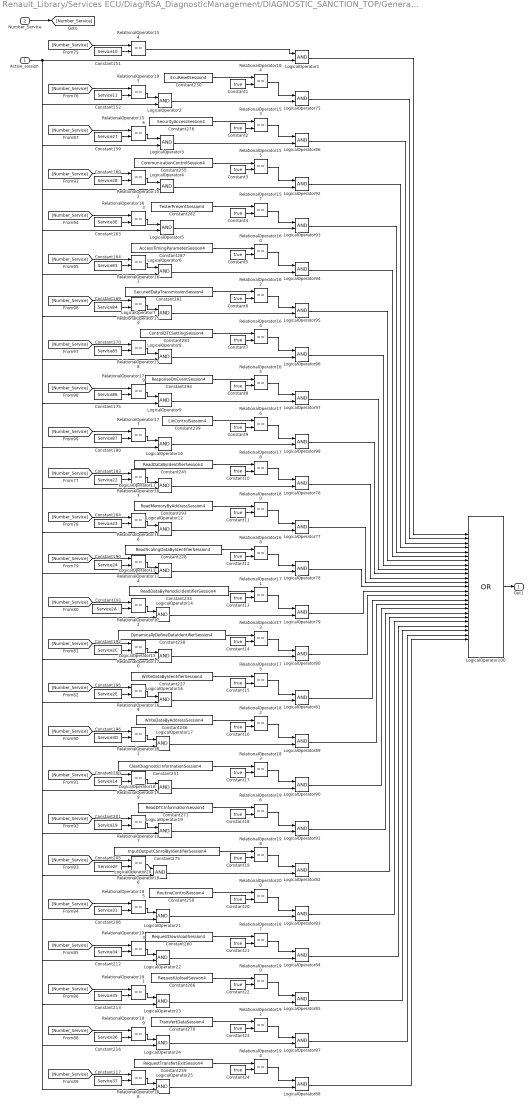
== system Renault_Library/Services ECU/Diag/RSA_DiagnosticManagement/DIAGNOSTIC_SANCTION_TOP/GeneralService/Service_Supported_In_Active_Session/SubsystemSafetySession ==
  Active_session : Inport
  Number_Service : Inport  Port=2
  Constant{1..24} (24 alike) : Constant  Value=true  OutDataTypeStr=boolean  SampleTime=-1
  Constant151 : Constant  Value=Service10  OutDataTypeStr=Inherit: Inherit via back propagation  SampleTime=-1
  Constant152 : Constant  Value=Service11  OutDataTypeStr=Inherit: Inherit via back propagation  SampleTime=-1
  Constant159 : Constant  Value=Service27  OutDataTypeStr=Inherit: Inherit via back propagation  SampleTime=-1
  Constant160 : Constant  Value=Service28  OutDataTypeStr=Inherit: Inherit via back propagation  SampleTime=-1
  Constant163 : Constant  Value=Service3E  OutDataTypeStr=Inherit: Inherit via back propagation  SampleTime=-1
  Constant164 : Constant  Value=Service83  OutDataTypeStr=Inherit: Inherit via back propagation  SampleTime=-1
  Constant169 : Constant  Value=Service84  OutDataTypeStr=Inherit: Inherit via back propagation  SampleTime=-1
  Constant170 : Constant  Value=Service85  OutDataTypeStr=Inherit: Inherit via back propagation  SampleTime=-1
  Constant175 : Constant  Value=Service86  OutDataTypeStr=Inherit: Inherit via back propagation  SampleTime=-1
  Constant180 : Constant  Value=Service87  OutDataTypeStr=Inherit: Inherit via back propagation  SampleTime=-1
  Constant183 : Constant  Value=Service22  OutDataTypeStr=Inherit: Inherit via back propagation  SampleTime=-1
  Constant184 : Constant  Value=Service23  OutDataTypeStr=Inherit: Inherit via back propagation  SampleTime=-1
  Constant190 : Constant  Value=Service24  OutDataTypeStr=Inherit: Inherit via back propagation  SampleTime=-1
  Constant191 : Constant  Value=Service2A  OutDataTypeStr=Inherit: Inherit via back propagation  SampleTime=-1
  Constant192 : Constant  Value=Service2C  OutDataTypeStr=Inherit: Inherit via back propagation  SampleTime=-1
  Constant195 : Constant  Value=Service2E  OutDataTypeStr=Inherit: Inherit via back propagation  SampleTime=-1
  Constant196 : Constant  Value=Service3D  OutDataTypeStr=Inherit: Inherit via back propagation  SampleTime=-1
  Constant200 : Constant  Value=Service14  OutDataTypeStr=Inherit: Inherit via back propagation  SampleTime=-1
  Constant201 : Constant  Value=Service19  OutDataTypeStr=Inherit: Inherit via back propagation  SampleTime=-1
  Constant205 : Constant  Value=Service2F  OutDataTypeStr=Inherit: Inherit via back propagation  SampleTime=-1
  Constant206 : Constant  Value=Service31  OutDataTypeStr=Inherit: Inherit via back propagation  SampleTime=-1
  Constant212 : Constant  Value=Service34  OutDataTypeStr=Inherit: Inherit via back propagation  SampleTime=-1
  Constant213 : Constant  Value=Service35  OutDataTypeStr=Inherit: Inherit via back propagation  SampleTime=-1
  Constant216 : Constant  Value=Service36  OutDataTypeStr=Inherit: Inherit via back propagation  SampleTime=-1
  Constant217 : Constant  Value=Service37  OutDataTypeStr=Inherit: Inherit via back propagation  SampleTime=-1
  Constant227 : Constant  Value=WriteDataByIdentifierSession4  OutDataTypeStr=Inherit: Inherit via back propagation  SampleTime=-1
  Constant228 : Constant  Value=ReadScalingDataByIdentifierSession4  OutDataTypeStr=Inherit: Inherit via back propagation  SampleTime=-1
  Constant230 : Constant  Value=EcuResetSession4  OutDataTypeStr=Inherit: Inherit via back propagation  SampleTime=-1
  Constant234 : Constant  Value=ReadDataByPeriodicIdentifierSession4  OutDataTypeStr=Inherit: Inherit via back propagation  SampleTime=-1
  Constant238 : Constant  Value=DynamicallyDefineDataIdentifierSession4  OutDataTypeStr=Inherit: Inherit via back propagation  SampleTime=-1
  Constant239 : Constant  Value=LinControlSession4  OutDataTypeStr=Inherit: Inherit via back propagation  SampleTime=-1
  Constant245 : Constant  Value=ReadDataByIdentifierSession4  OutDataTypeStr=Inherit: Inherit via back propagation  SampleTime=-1
  Constant246 : Constant  Value=WriteDataByAddressSession4  OutDataTypeStr=Inherit: Inherit via back propagation  SampleTime=-1
  Constant250 : Constant  Value=RoutineControlSession4  OutDataTypeStr=Inherit: Inherit via back propagation  SampleTime=-1
  Constant251 : Constant  Value=ClearDiagnosticInformationSession4  OutDataTypeStr=Inherit: Inherit via back propagation  SampleTime=-1
  Constant255 : Constant  Value=CommunicationControlSession4  OutDataTypeStr=Inherit: Inherit via back propagation  SampleTime=-1
  Constant259 : Constant  Value=RequestTransfertExitSession4  OutDataTypeStr=Inherit: Inherit via back propagation  SampleTime=-1
  Constant260 : Constant  Value=RequestDownloadSession4  OutDataTypeStr=Inherit: Inherit via back propagation  SampleTime=-1
  Constant266 : Constant  Value=RequestUploadSession4  OutDataTypeStr=Inherit: Inherit via back propagation  SampleTime=-1
  Constant270 : Constant  Value=TransfertDataSession4  OutDataTypeStr=Inherit: Inherit via back propagation  SampleTime=-1
  Constant271 : Constant  Value=ReadDTCInformationSession4  OutDataTypeStr=Inherit: Inherit via back propagation  SampleTime=-1
  Constant275 : Constant  Value=InputOutputConrolByIdentifierSession4  OutDataTypeStr=Inherit: Inherit via back propagation  SampleTime=-1
  Constant276 : Constant  Value=SecurityAccessSession4  OutDataTypeStr=Inherit: Inherit via back propagation  SampleTime=-1
  Constant281 : Constant  Value=ControlDTCSettingSession4  OutDataTypeStr=Inherit: Inherit via back propagation  SampleTime=-1
  Constant282 : Constant  Value=TesterPresentSession4  OutDataTypeStr=Inherit: Inherit via back propagation  SampleTime=-1
  Constant287 : Constant  Value=AccessTimingParameterSession4  OutDataTypeStr=Inherit: Inherit via back propagation  SampleTime=-1
  Constant291 : Constant  Value=SecuredDataTransmissionSession4  OutDataTypeStr=Inherit: Inherit via back propagation  SampleTime=-1
  Constant293 : Constant  Value=ReadMemoryByAddressSession4  OutDataTypeStr=Inherit: Inherit via back propagation  SampleTime=-1
  Constant294 : Constant  Value=ResponseOnEventSession4  OutDataTypeStr=Inherit: Inherit via back propagation  SampleTime=-1
  LogicalOperator{1..25,75..98} (49 alike) : Logic  OutDataTypeStr=boolean
  LogicalOperator100 : Logic  Operator=OR  Inputs=25  OutDataTypeStr=boolean
  RelationalOperator{152..200} (49 alike) : RelationalOperator  Operator===  OutDataTypeStr=boolean  RndMeth=Nearest  InputSameDT=on
  Out1 : Outport
  wires:
  Constant151/1 -> RelationalOperator154/2
  Active_session/1 -> LogicalOperator17/2, LogicalOperator18/2, LogicalOperator19/2, LogicalOperator20/2, LogicalOperator21/2, LogicalOperator22/2, LogicalOperator23/2, LogicalOperator24/2, LogicalOperator25/2, LogicalOperator9/2, LogicalOperator10/2, LogicalOperator11/2, LogicalOperator12/2, LogicalOperator13/2, LogicalOperator14/2, LogicalOperator15/2, LogicalOperator16/2, LogicalOperator1/2, LogicalOperator2/2, LogicalOperator3/2, LogicalOperator4/2, LogicalOperator5/2, LogicalOperator6/2, LogicalOperator7/2, LogicalOperator8/2
  RelationalOperator154/1 -> LogicalOperator1/1
  [Number_Service] -> RelationalOperator154/1
  Constant152/1 -> RelationalOperator195/2
  Constant230/1 -> RelationalOperator184/1
  RelationalOperator184/1 -> LogicalOperator75/1
  LogicalOperator2/1 -> LogicalOperator75/2
  RelationalOperator195/1 -> LogicalOperator2/1
  [Number_Service] -> RelationalOperator195/1
  Constant159/1 -> RelationalOperator156/2
  Constant276/1 -> RelationalOperator153/1
  RelationalOperator153/1 -> LogicalOperator86/1
  LogicalOperator3/1 -> LogicalOperator86/2
  RelationalOperator156/1 -> LogicalOperator3/1
  [Number_Service] -> RelationalOperator156/1
  Constant160/1 -> RelationalOperator152/2
  Constant255/1 -> RelationalOperator155/1
  Constant3/1 -> RelationalOperator155/2
  RelationalOperator155/1 -> LogicalOperator92/1
  LogicalOperator4/1 -> LogicalOperator92/2
  RelationalOperator152/1 -> LogicalOperator4/1
  [Number_Service] -> RelationalOperator152/1
  Constant163/1 -> RelationalOperator163/2
  Constant282/1 -> RelationalOperator157/1
  RelationalOperator157/1 -> LogicalOperator93/1
  LogicalOperator5/1 -> LogicalOperator93/2
  RelationalOperator163/1 -> LogicalOperator5/1
  [Number_Service] -> RelationalOperator163/1
  Constant164/1 -> RelationalOperator161/2
  Constant287/1 -> RelationalOperator160/1
  Constant5/1 -> RelationalOperator160/2
  RelationalOperator160/1 -> LogicalOperator94/1
  LogicalOperator6/1 -> LogicalOperator94/2
  RelationalOperator161/1 -> LogicalOperator6/1
  [Number_Service] -> RelationalOperator161/1
  Constant169/1 -> RelationalOperator159/2
  Constant291/1 -> RelationalOperator162/1
  Constant6/1 -> RelationalOperator162/2
  RelationalOperator162/1 -> LogicalOperator95/1
  LogicalOperator7/1 -> LogicalOperator95/2
  RelationalOperator159/1 -> LogicalOperator7/1
  [Number_Service] -> RelationalOperator159/1
  Constant170/1 -> RelationalOperator158/2
  Constant281/1 -> RelationalOperator164/1
  Constant7/1 -> RelationalOperator164/2
  RelationalOperator164/1 -> LogicalOperator96/1
  LogicalOperator8/1 -> LogicalOperator96/2
  RelationalOperator158/1 -> LogicalOperator8/1
  [Number_Service] -> RelationalOperator158/1
  Constant175/1 -> RelationalOperator179/2
  Constant294/1 -> RelationalOperator165/1
  Constant8/1 -> RelationalOperator165/2
  RelationalOperator165/1 -> LogicalOperator97/1
  LogicalOperator9/1 -> LogicalOperator97/2
  RelationalOperator179/1 -> LogicalOperator9/1
  [Number_Service] -> RelationalOperator179/1
  Constant180/1 -> RelationalOperator177/2
  Constant239/1 -> RelationalOperator176/1
  Constant9/1 -> RelationalOperator176/2
  RelationalOperator176/1 -> LogicalOperator98/1
  LogicalOperator10/1 -> LogicalOperator98/2
  RelationalOperator177/1 -> LogicalOperator10/1
  [Number_Service] -> RelationalOperator177/1
  Constant183/1 -> RelationalOperator167/2
  Constant245/1 -> RelationalOperator178/1
  Constant10/1 -> RelationalOperator178/2
  RelationalOperator178/1 -> LogicalOperator76/1
  LogicalOperator11/1 -> LogicalOperator76/2
  RelationalOperator167/1 -> LogicalOperator11/1
  [Number_Service] -> RelationalOperator167/1
  Constant184/1 -> RelationalOperator166/2
  Constant293/1 -> RelationalOperator180/1
  RelationalOperator180/1 -> LogicalOperator77/1
  LogicalOperator12/1 -> LogicalOperator77/2
  RelationalOperator166/1 -> LogicalOperator12/1
  [Number_Service] -> RelationalOperator166/1
  Constant190/1 -> RelationalOperator174/2
  Constant228/1 -> RelationalOperator168/1
  Constant12/1 -> RelationalOperator168/2
  RelationalOperator168/1 -> LogicalOperator78/1
  LogicalOperator13/1 -> LogicalOperator78/2
  RelationalOperator174/1 -> LogicalOperator13/1
  [Number_Service] -> RelationalOperator174/1
  Constant191/1 -> RelationalOperator172/2
  Constant234/1 -> RelationalOperator171/1
  Constant13/1 -> RelationalOperator171/2
  RelationalOperator171/1 -> LogicalOperator79/1
  LogicalOperator14/1 -> LogicalOperator79/2
  RelationalOperator172/1 -> LogicalOperator14/1
  [Number_Service] -> RelationalOperator172/1
  Constant192/1 -> RelationalOperator170/2
  Constant238/1 -> RelationalOperator173/1
  Constant14/1 -> RelationalOperator173/2
  RelationalOperator173/1 -> LogicalOperator80/1
  LogicalOperator15/1 -> LogicalOperator80/2
  RelationalOperator170/1 -> LogicalOperator15/1
  [Number_Service] -> RelationalOperator170/1
  Constant195/1 -> RelationalOperator169/2
  Constant227/1 -> RelationalOperator175/1
  Constant15/1 -> RelationalOperator175/2
  RelationalOperator175/1 -> LogicalOperator81/1
  LogicalOperator16/1 -> LogicalOperator81/2
  RelationalOperator169/1 -> LogicalOperator16/1
  [Number_Service] -> RelationalOperator169/1
  Constant196/1 -> RelationalOperator181/2
  Constant246/1 -> RelationalOperator182/1
  Constant16/1 -> RelationalOperator182/2
  RelationalOperator182/1 -> LogicalOperator89/1
  LogicalOperator17/1 -> LogicalOperator89/2
  RelationalOperator181/1 -> LogicalOperator17/1
  [Number_Service] -> RelationalOperator181/1
  Constant200/1 -> RelationalOperator199/2
  Constant251/1 -> RelationalOperator183/1
  Constant17/1 -> RelationalOperator183/2
  RelationalOperator183/1 -> LogicalOperator90/1
  LogicalOperator18/1 -> LogicalOperator90/2
  RelationalOperator199/1 -> LogicalOperator18/1
  [Number_Service] -> RelationalOperator199/1
  Constant201/1 -> RelationalOperator197/2
  Constant271/1 -> RelationalOperator196/1
  Constant18/1 -> RelationalOperator196/2
  RelationalOperator196/1 -> LogicalOperator91/1
  LogicalOperator19/1 -> LogicalOperator91/2
  RelationalOperator197/1 -> LogicalOperator19/1
  [Number_Service] -> RelationalOperator197/1
  Constant205/1 -> RelationalOperator186/2
  Constant275/1 -> RelationalOperator198/1
  Constant19/1 -> RelationalOperator198/2
  RelationalOperator198/1 -> LogicalOperator82/1
  LogicalOperator20/1 -> LogicalOperator82/2
  RelationalOperator186/1 -> LogicalOperator20/1
  [Number_Service] -> RelationalOperator186/1
  Constant206/1 -> RelationalOperator185/2
  Constant250/1 -> RelationalOperator200/1
  Constant20/1 -> RelationalOperator200/2
  RelationalOperator200/1 -> LogicalOperator83/1
  LogicalOperator21/1 -> LogicalOperator83/2
  RelationalOperator185/1 -> LogicalOperator21/1
  [Number_Service] -> RelationalOperator185/1
  Constant212/1 -> RelationalOperator193/2
  Constant260/1 -> RelationalOperator187/1
  Constant21/1 -> RelationalOperator187/2
  RelationalOperator187/1 -> LogicalOperator84/1
  LogicalOperator22/1 -> LogicalOperator84/2
  RelationalOperator193/1 -> LogicalOperator22/1
  [Number_Service] -> RelationalOperator193/1
  Constant213/1 -> RelationalOperator191/2
  Constant266/1 -> RelationalOperator190/1
  Constant22/1 -> RelationalOperator190/2
  RelationalOperator190/1 -> LogicalOperator85/1
  LogicalOperator23/1 -> LogicalOperator85/2
  RelationalOperator191/1 -> LogicalOperator23/1
  [Number_Service] -> RelationalOperator191/1
  Constant216/1 -> RelationalOperator189/2
  Constant270/1 -> RelationalOperator192/1
  Constant23/1 -> RelationalOperator192/2
  RelationalOperator192/1 -> LogicalOperator87/1
  LogicalOperator24/1 -> LogicalOperator87/2
  RelationalOperator189/1 -> LogicalOperator24/1
  [Number_Service] -> RelationalOperator189/1
  Constant259/1 -> RelationalOperator194/1
  Constant24/1 -> RelationalOperator194/2
  RelationalOperator194/1 -> LogicalOperator88/1
  LogicalOperator25/1 -> LogicalOperator88/2
  LogicalOperator1/1 -> LogicalOperator100/1
  LogicalOperator75/1 -> LogicalOperator100/2
  LogicalOperator86/1 -> LogicalOperator100/3
  LogicalOperator92/1 -> LogicalOperator100/4
  LogicalOperator93/1 -> LogicalOperator100/5
  LogicalOperator94/1 -> LogicalOperator100/6
  LogicalOperator95/1 -> LogicalOperator100/7
  LogicalOperator96/1 -> LogicalOperator100/8
  LogicalOperator97/1 -> LogicalOperator100/9
  LogicalOperator98/1 -> LogicalOperator100/10
  LogicalOperator76/1 -> LogicalOperator100/11
  LogicalOperator77/1 -> LogicalOperator100/12
  LogicalOperator78/1 -> LogicalOperator100/13
  LogicalOperator79/1 -> LogicalOperator100/14
  LogicalOperator80/1 -> LogicalOperator100/15
  LogicalOperator81/1 -> LogicalOperator100/16
  LogicalOperator89/1 -> LogicalOperator100/17
  LogicalOperator90/1 -> LogicalOperator100/18
  LogicalOperator91/1 -> LogicalOperator100/19
  LogicalOperator82/1 -> LogicalOperator100/20
  LogicalOperator83/1 -> LogicalOperator100/21
  LogicalOperator84/1 -> LogicalOperator100/22
  LogicalOperator85/1 -> LogicalOperator100/23
  LogicalOperator87/1 -> LogicalOperator100/24
  LogicalOperator88/1 -> LogicalOperator100/25
  [Number_Service] -> RelationalOperator188/1
  Constant217/1 -> RelationalOperator188/2
  RelationalOperator188/1 -> LogicalOperator25/1
  LogicalOperator100/1 -> Out1/1
  Number_Service/1 -> [Number_Service]
  Constant11/1 -> RelationalOperator180/2
  Constant2/1 -> RelationalOperator153/2
  Constant1/1 -> RelationalOperator184/2
  Constant4/1 -> RelationalOperator157/2
